simulink model "complex_iq_bw2048_out32_fixaxi_v12b"  (R2019a) (continued)
== system complex_iq_bw2048_out32_fixaxi_v12b/dec_fir1 == (continued)
  fir_dbl_col2/22(imag_back_out3) -> fir_dbl_col1/22(imag_back3)
  fir_dbl_col2/23(real_back_out4) -> fir_dbl_col1/23(real_back4)
  fir_dbl_col2/24(imag_back_out4) -> fir_dbl_col1/24(imag_back4)
  fir_dbl_col2/25(real_back_out5) -> fir_dbl_col1/25(real_back5)
  fir_dbl_col2/26(imag_back_out5) -> fir_dbl_col1/26(imag_back5)
  fir_dbl_col2/27(real_back_out6) -> fir_dbl_col1/27(real_back6)
  fir_dbl_col2/28(imag_back_out6) -> fir_dbl_col1/28(imag_back6)
  fir_dbl_col2/29(real_back_out7) -> fir_dbl_col1/29(real_back7)
  fir_dbl_col2/30(imag_back_out7) -> fir_dbl_col1/30(imag_back7)
  fir_dbl_col2/31(real_back_out8) -> fir_dbl_col1/31(real_back8)
  fir_dbl_col2/32(imag_back_out8) -> fir_dbl_col1/32(imag_back8)
  fir_dbl_col3/17(real_back_out1) -> fir_dbl_col2/17(real_back1)
  fir_dbl_col3/18(imag_back_out1) -> fir_dbl_col2/18(imag_back1)
  fir_dbl_col3/19(real_back_out2) -> fir_dbl_col2/19(real_back2)
  fir_dbl_col3/20(imag_back_out2) -> fir_dbl_col2/20(imag_back2)
  fir_dbl_col3/21(real_back_out3) -> fir_dbl_col2/21(real_back3)
  fir_dbl_col3/22(imag_back_out3) -> fir_dbl_col2/22(imag_back3)
  fir_dbl_col3/23(real_back_out4) -> fir_dbl_col2/23(real_back4)
  fir_dbl_col3/24(imag_back_out4) -> fir_dbl_col2/24(imag_back4)
  fir_dbl_col3/25(real_back_out5) -> fir_dbl_col2/25(real_back5)
  fir_dbl_col3/26(imag_back_out5) -> fir_dbl_col2/26(imag_back5)
  fir_dbl_col3/27(real_back_out6) -> fir_dbl_col2/27(real_back6)
  fir_dbl_col3/28(imag_back_out6) -> fir_dbl_col2/28(imag_back6)
  fir_dbl_col3/29(real_back_out7) -> fir_dbl_col2/29(real_back7)
  fir_dbl_col3/30(imag_back_out7) -> fir_dbl_col2/30(imag_back7)
  fir_dbl_col3/31(real_back_out8) -> fir_dbl_col2/31(real_back8)
  fir_dbl_col3/32(imag_back_out8) -> fir_dbl_col2/32(imag_back8)
  fir_dbl_col4/17(real_back_out1) -> fir_dbl_col3/17(real_back1)
  fir_dbl_col4/18(imag_back_out1) -> fir_dbl_col3/18(imag_back1)
  fir_dbl_col4/19(real_back_out2) -> fir_dbl_col3/19(real_back2)
  fir_dbl_col4/20(imag_back_out2) -> fir_dbl_col3/20(imag_back2)
  fir_dbl_col4/21(real_back_out3) -> fir_dbl_col3/21(real_back3)
  fir_dbl_col4/22(imag_back_out3) -> fir_dbl_col3/22(imag_back3)
  fir_dbl_col4/23(real_back_out4) -> fir_dbl_col3/23(real_back4)
  fir_dbl_col4/24(imag_back_out4) -> fir_dbl_col3/24(imag_back4)
  fir_dbl_col4/25(real_back_out5) -> fir_dbl_col3/25(real_back5)
  fir_dbl_col4/26(imag_back_out5) -> fir_dbl_col3/26(imag_back5)
  fir_dbl_col4/27(real_back_out6) -> fir_dbl_col3/27(real_back6)
  fir_dbl_col4/28(imag_back_out6) -> fir_dbl_col3/28(imag_back6)
  fir_dbl_col4/29(real_back_out7) -> fir_dbl_col3/29(real_back7)
  fir_dbl_col4/30(imag_back_out7) -> fir_dbl_col3/30(imag_back7)
  fir_dbl_col4/31(real_back_out8) -> fir_dbl_col3/31(real_back8)
  fir_dbl_col4/32(imag_back_out8) -> fir_dbl_col3/32(imag_back8)
  fir_dbl_col5/17(real_back_out1) -> fir_dbl_col4/17(real_back1)
  fir_dbl_col5/18(imag_back_out1) -> fir_dbl_col4/18(imag_back1)
  fir_dbl_col5/19(real_back_out2) -> fir_dbl_col4/19(real_back2)
  fir_dbl_col5/20(imag_back_out2) -> fir_dbl_col4/20(imag_back2)
  fir_dbl_col5/21(real_back_out3) -> fir_dbl_col4/21(real_back3)
  fir_dbl_col5/22(imag_back_out3) -> fir_dbl_col4/22(imag_back3)
  fir_dbl_col5/23(real_back_out4) -> fir_dbl_col4/23(real_back4)
  fir_dbl_col5/24(imag_back_out4) -> fir_dbl_col4/24(imag_back4)
  fir_dbl_col5/25(real_back_out5) -> fir_dbl_col4/25(real_back5)
  fir_dbl_col5/26(imag_back_out5) -> fir_dbl_col4/26(imag_back5)
  fir_dbl_col5/27(real_back_out6) -> fir_dbl_col4/27(real_back6)
  fir_dbl_col5/28(imag_back_out6) -> fir_dbl_col4/28(imag_back6)
  fir_dbl_col5/29(real_back_out7) -> fir_dbl_col4/29(real_back7)
  fir_dbl_col5/30(imag_back_out7) -> fir_dbl_col4/30(imag_back7)
  fir_dbl_col5/31(real_back_out8) -> fir_dbl_col4/31(real_back8)
  fir_dbl_col5/32(imag_back_out8) -> fir_dbl_col4/32(imag_back8)
  fir_dbl_col6/17(real_back_out1) -> fir_dbl_col5/17(real_back1)
  fir_dbl_col6/18(imag_back_out1) -> fir_dbl_col5/18(imag_back1)
  fir_dbl_col6/19(real_back_out2) -> fir_dbl_col5/19(real_back2)
  fir_dbl_col6/20(imag_back_out2) -> fir_dbl_col5/20(imag_back2)
  fir_dbl_col6/21(real_back_out3) -> fir_dbl_col5/21(real_back3)
  fir_dbl_col6/22(imag_back_out3) -> fir_dbl_col5/22(imag_back3)
  fir_dbl_col6/23(real_back_out4) -> fir_dbl_col5/23(real_back4)
  fir_dbl_col6/24(imag_back_out4) -> fir_dbl_col5/24(imag_back4)
  fir_dbl_col6/25(real_back_out5) -> fir_dbl_col5/25(real_back5)
  fir_dbl_col6/26(imag_back_out5) -> fir_dbl_col5/26(imag_back5)
  fir_dbl_col6/27(real_back_out6) -> fir_dbl_col5/27(real_back6)
  fir_dbl_col6/28(imag_back_out6) -> fir_dbl_col5/28(imag_back6)
  fir_dbl_col6/29(real_back_out7) -> fir_dbl_col5/29(real_back7)
  fir_dbl_col6/30(imag_back_out7) -> fir_dbl_col5/30(imag_back7)
  fir_dbl_col6/31(real_back_out8) -> fir_dbl_col5/31(real_back8)
  fir_dbl_col6/32(imag_back_out8) -> fir_dbl_col5/32(imag_back8)
  fir_dbl_col7/17(real_back_out1) -> fir_dbl_col6/17(real_back1)
  fir_dbl_col7/18(imag_back_out1) -> fir_dbl_col6/18(imag_back1)
  fir_dbl_col7/19(real_back_out2) -> fir_dbl_col6/19(real_back2)
  fir_dbl_col7/20(imag_back_out2) -> fir_dbl_col6/20(imag_back2)
  fir_dbl_col7/21(real_back_out3) -> fir_dbl_col6/21(real_back3)
  fir_dbl_col7/22(imag_back_out3) -> fir_dbl_col6/22(imag_back3)
  fir_dbl_col7/23(real_back_out4) -> fir_dbl_col6/23(real_back4)
  fir_dbl_col7/24(imag_back_out4) -> fir_dbl_col6/24(imag_back4)
  fir_dbl_col7/25(real_back_out5) -> fir_dbl_col6/25(real_back5)
  fir_dbl_col7/26(imag_back_out5) -> fir_dbl_col6/26(imag_back5)
  fir_dbl_col7/27(real_back_out6) -> fir_dbl_col6/27(real_back6)
  fir_dbl_col7/28(imag_back_out6) -> fir_dbl_col6/28(imag_back6)
  fir_dbl_col7/29(real_back_out7) -> fir_dbl_col6/29(real_back7)
  fir_dbl_col7/30(imag_back_out7) -> fir_dbl_col6/30(imag_back7)
  fir_dbl_col7/31(real_back_out8) -> fir_dbl_col6/31(real_back8)
  fir_dbl_col7/32(imag_back_out8) -> fir_dbl_col6/32(imag_back8)
  fir_dbl_col8/17(real_back_out1) -> fir_dbl_col7/17(real_back1)
  fir_dbl_col8/18(imag_back_out1) -> fir_dbl_col7/18(imag_back1)
  fir_dbl_col8/19(real_back_out2) -> fir_dbl_col7/19(real_back2)
  fir_dbl_col8/20(imag_back_out2) -> fir_dbl_col7/20(imag_back2)
  fir_dbl_col8/21(real_back_out3) -> fir_dbl_col7/21(real_back3)
  fir_dbl_col8/22(imag_back_out3) -> fir_dbl_col7/22(imag_back3)
  fir_dbl_col8/23(real_back_out4) -> fir_dbl_col7/23(real_back4)
  fir_dbl_col8/24(imag_back_out4) -> fir_dbl_col7/24(imag_back4)
  fir_dbl_col8/25(real_back_out5) -> fir_dbl_col7/25(real_back5)
  fir_dbl_col8/26(imag_back_out5) -> fir_dbl_col7/26(imag_back5)
  fir_dbl_col8/27(real_back_out6) -> fir_dbl_col7/27(real_back6)
  fir_dbl_col8/28(imag_back_out6) -> fir_dbl_col7/28(imag_back6)
  fir_dbl_col8/29(real_back_out7) -> fir_dbl_col7/29(real_back7)
  fir_dbl_col8/30(imag_back_out7) -> fir_dbl_col7/30(imag_back7)
  fir_dbl_col8/31(real_back_out8) -> fir_dbl_col7/31(real_back8)
  fir_dbl_col8/32(imag_back_out8) -> fir_dbl_col7/32(imag_back8)
  fir_dbl_col9/17(real_back_out1) -> fir_dbl_col8/17(real_back1)
  fir_dbl_col9/18(imag_back_out1) -> fir_dbl_col8/18(imag_back1)
  fir_dbl_col9/19(real_back_out2) -> fir_dbl_col8/19(real_back2)
  fir_dbl_col9/20(imag_back_out2) -> fir_dbl_col8/20(imag_back2)
  fir_dbl_col9/21(real_back_out3) -> fir_dbl_col8/21(real_back3)
  fir_dbl_col9/22(imag_back_out3) -> fir_dbl_col8/22(imag_back3)
  fir_dbl_col9/23(real_back_out4) -> fir_dbl_col8/23(real_back4)
  fir_dbl_col9/24(imag_back_out4) -> fir_dbl_col8/24(imag_back4)
  fir_dbl_col9/25(real_back_out5) -> fir_dbl_col8/25(real_back5)
  fir_dbl_col9/26(imag_back_out5) -> fir_dbl_col8/26(imag_back5)
  fir_dbl_col9/27(real_back_out6) -> fir_dbl_col8/27(real_back6)
  fir_dbl_col9/28(imag_back_out6) -> fir_dbl_col8/28(imag_back6)
  fir_dbl_col9/29(real_back_out7) -> fir_dbl_col8/29(real_back7)
  fir_dbl_col9/30(imag_back_out7) -> fir_dbl_col8/30(imag_back7)
  fir_dbl_col9/31(real_back_out8) -> fir_dbl_col8/31(real_back8)
  fir_dbl_col9/32(imag_back_out8) -> fir_dbl_col8/32(imag_back8)
  fir_dbl_col10/17(real_back_out1) -> fir_dbl_col9/17(real_back1)
  fir_dbl_col10/18(imag_back_out1) -> fir_dbl_col9/18(imag_back1)
  fir_dbl_col10/19(real_back_out2) -> fir_dbl_col9/19(real_back2)
  fir_dbl_col10/20(imag_back_out2) -> fir_dbl_col9/20(imag_back2)
  fir_dbl_col10/21(real_back_out3) -> fir_dbl_col9/21(real_back3)
  fir_dbl_col10/22(imag_back_out3) -> fir_dbl_col9/22(imag_back3)
  fir_dbl_col10/23(real_back_out4) -> fir_dbl_col9/23(real_back4)
  fir_dbl_col10/24(imag_back_out4) -> fir_dbl_col9/24(imag_back4)
  fir_dbl_col10/25(real_back_out5) -> fir_dbl_col9/25(real_back5)
  fir_dbl_col10/26(imag_back_out5) -> fir_dbl_col9/26(imag_back5)
  fir_dbl_col10/27(real_back_out6) -> fir_dbl_col9/27(real_back6)
  fir_dbl_col10/28(imag_back_out6) -> fir_dbl_col9/28(imag_back6)
  fir_dbl_col10/29(real_back_out7) -> fir_dbl_col9/29(real_back7)
  fir_dbl_col10/30(imag_back_out7) -> fir_dbl_col9/30(imag_back7)
  fir_dbl_col10/31(real_back_out8) -> fir_dbl_col9/31(real_back8)
  fir_dbl_col10/32(imag_back_out8) -> fir_dbl_col9/32(imag_back8)
  fir_dbl_col11/17(real_back_out1) -> fir_dbl_col10/17(real_back1)
  fir_dbl_col11/18(imag_back_out1) -> fir_dbl_col10/18(imag_back1)
  fir_dbl_col11/19(real_back_out2) -> fir_dbl_col10/19(real_back2)
  fir_dbl_col11/20(imag_back_out2) -> fir_dbl_col10/20(imag_back2)
  fir_dbl_col11/21(real_back_out3) -> fir_dbl_col10/21(real_back3)
  fir_dbl_col11/22(imag_back_out3) -> fir_dbl_col10/22(imag_back3)
  fir_dbl_col11/23(real_back_out4) -> fir_dbl_col10/23(real_back4)
  fir_dbl_col11/24(imag_back_out4) -> fir_dbl_col10/24(imag_back4)
  fir_dbl_col11/25(real_back_out5) -> fir_dbl_col10/25(real_back5)
  fir_dbl_col11/26(imag_back_out5) -> fir_dbl_col10/26(imag_back5)
  fir_dbl_col11/27(real_back_out6) -> fir_dbl_col10/27(real_back6)
  fir_dbl_col11/28(imag_back_out6) -> fir_dbl_col10/28(imag_back6)
  fir_dbl_col11/29(real_back_out7) -> fir_dbl_col10/29(real_back7)
  fir_dbl_col11/30(imag_back_out7) -> fir_dbl_col10/30(imag_back7)
  fir_dbl_col11/31(real_back_out8) -> fir_dbl_col10/31(real_back8)
  fir_dbl_col11/32(imag_back_out8) -> fir_dbl_col10/32(imag_back8)
  fir_dbl_col12/17(real_back_out1) -> fir_dbl_col11/17(real_back1)
  fir_dbl_col12/18(imag_back_out1) -> fir_dbl_col11/18(imag_back1)
  fir_dbl_col12/19(real_back_out2) -> fir_dbl_col11/19(real_back2)
  fir_dbl_col12/20(imag_back_out2) -> fir_dbl_col11/20(imag_back2)
  fir_dbl_col12/21(real_back_out3) -> fir_dbl_col11/21(real_back3)
  fir_dbl_col12/22(imag_back_out3) -> fir_dbl_col11/22(imag_back3)
  fir_dbl_col12/23(real_back_out4) -> fir_dbl_col11/23(real_back4)
  fir_dbl_col12/24(imag_back_out4) -> fir_dbl_col11/24(imag_back4)
  fir_dbl_col12/25(real_back_out5) -> fir_dbl_col11/25(real_back5)
  fir_dbl_col12/26(imag_back_out5) -> fir_dbl_col11/26(imag_back5)
  fir_dbl_col12/27(real_back_out6) -> fir_dbl_col11/27(real_back6)
  fir_dbl_col12/28(imag_back_out6) -> fir_dbl_col11/28(imag_back6)
  fir_dbl_col12/29(real_back_out7) -> fir_dbl_col11/29(real_back7)
  fir_dbl_col12/30(imag_back_out7) -> fir_dbl_col11/30(imag_back7)
  fir_dbl_col12/31(real_back_out8) -> fir_dbl_col11/31(real_back8)
  fir_dbl_col12/32(imag_back_out8) -> fir_dbl_col11/32(imag_back8)
  fir_dbl_col13/17(real_back_out1) -> fir_dbl_col12/17(real_back1)
  fir_dbl_col13/18(imag_back_out1) -> fir_dbl_col12/18(imag_back1)
  fir_dbl_col13/19(real_back_out2) -> fir_dbl_col12/19(real_back2)
  fir_dbl_col13/20(imag_back_out2) -> fir_dbl_col12/20(imag_back2)
  fir_dbl_col13/21(real_back_out3) -> fir_dbl_col12/21(real_back3)
  fir_dbl_col13/22(imag_back_out3) -> fir_dbl_col12/22(imag_back3)
  fir_dbl_col13/23(real_back_out4) -> fir_dbl_col12/23(real_back4)
  fir_dbl_col13/24(imag_back_out4) -> fir_dbl_col12/24(imag_back4)
  fir_dbl_col13/25(real_back_out5) -> fir_dbl_col12/25(real_back5)
  fir_dbl_col13/26(imag_back_out5) -> fir_dbl_col12/26(imag_back5)
  fir_dbl_col13/27(real_back_out6) -> fir_dbl_col12/27(real_back6)
  fir_dbl_col13/28(imag_back_out6) -> fir_dbl_col12/28(imag_back6)
  fir_dbl_col13/29(real_back_out7) -> fir_dbl_col12/29(real_back7)
  fir_dbl_col13/30(imag_back_out7) -> fir_dbl_col12/30(imag_back7)
  fir_dbl_col13/31(real_back_out8) -> fir_dbl_col12/31(real_back8)
  fir_dbl_col13/32(imag_back_out8) -> fir_dbl_col12/32(imag_back8)
  fir_dbl_col14/17(real_back_out1) -> fir_dbl_col13/17(real_back1)
  fir_dbl_col14/18(imag_back_out1) -> fir_dbl_col13/18(imag_back1)
  fir_dbl_col14/19(real_back_out2) -> fir_dbl_col13/19(real_back2)
  fir_dbl_col14/20(imag_back_out2) -> fir_dbl_col13/20(imag_back2)
  fir_dbl_col14/21(real_back_out3) -> fir_dbl_col13/21(real_back3)
  fir_dbl_col14/22(imag_back_out3) -> fir_dbl_col13/22(imag_back3)
  fir_dbl_col14/23(real_back_out4) -> fir_dbl_col13/23(real_back4)
  fir_dbl_col14/24(imag_back_out4) -> fir_dbl_col13/24(imag_back4)
  fir_dbl_col14/25(real_back_out5) -> fir_dbl_col13/25(real_back5)
  fir_dbl_col14/26(imag_back_out5) -> fir_dbl_col13/26(imag_back5)
  fir_dbl_col14/27(real_back_out6) -> fir_dbl_col13/27(real_back6)
  fir_dbl_col14/28(imag_back_out6) -> fir_dbl_col13/28(imag_back6)
  fir_dbl_col14/29(real_back_out7) -> fir_dbl_col13/29(real_back7)
  fir_dbl_col14/30(imag_back_out7) -> fir_dbl_col13/30(imag_back7)
  fir_dbl_col14/31(real_back_out8) -> fir_dbl_col13/31(real_back8)
  fir_dbl_col14/32(imag_back_out8) -> fir_dbl_col13/32(imag_back8)
  fir_dbl_col15/17(real_back_out1) -> fir_dbl_col14/17(real_back1)
  fir_dbl_col15/18(imag_back_out1) -> fir_dbl_col14/18(imag_back1)
  fir_dbl_col15/19(real_back_out2) -> fir_dbl_col14/19(real_back2)
  fir_dbl_col15/20(imag_back_out2) -> fir_dbl_col14/20(imag_back2)
  fir_dbl_col15/21(real_back_out3) -> fir_dbl_col14/21(real_back3)
  fir_dbl_col15/22(imag_back_out3) -> fir_dbl_col14/22(imag_back3)
  fir_dbl_col15/23(real_back_out4) -> fir_dbl_col14/23(real_back4)
  fir_dbl_col15/24(imag_back_out4) -> fir_dbl_col14/24(imag_back4)
  fir_dbl_col15/25(real_back_out5) -> fir_dbl_col14/25(real_back5)
  fir_dbl_col15/26(imag_back_out5) -> fir_dbl_col14/26(imag_back5)
  fir_dbl_col15/27(real_back_out6) -> fir_dbl_col14/27(real_back6)
  fir_dbl_col15/28(imag_back_out6) -> fir_dbl_col14/28(imag_back6)
  fir_dbl_col15/29(real_back_out7) -> fir_dbl_col14/29(real_back7)
  fir_dbl_col15/30(imag_back_out7) -> fir_dbl_col14/30(imag_back7)
  fir_dbl_col15/31(real_back_out8) -> fir_dbl_col14/31(real_back8)
  fir_dbl_col15/32(imag_back_out8) -> fir_dbl_col14/32(imag_back8)
  fir_dbl_col16/17(real_back_out1) -> fir_dbl_col15/17(real_back1)
  fir_dbl_col16/18(imag_back_out1) -> fir_dbl_col15/18(imag_back1)
  fir_dbl_col16/19(real_back_out2) -> fir_dbl_col15/19(real_back2)
  fir_dbl_col16/20(imag_back_out2) -> fir_dbl_col15/20(imag_back2)
  fir_dbl_col16/21(real_back_out3) -> fir_dbl_col15/21(real_back3)
  fir_dbl_col16/22(imag_back_out3) -> fir_dbl_col15/22(imag_back3)
  fir_dbl_col16/23(real_back_out4) -> fir_dbl_col15/23(real_back4)
  fir_dbl_col16/24(imag_back_out4) -> fir_dbl_col15/24(imag_back4)
  fir_dbl_col16/25(real_back_out5) -> fir_dbl_col15/25(real_back5)
  fir_dbl_col16/26(imag_back_out5) -> fir_dbl_col15/26(imag_back5)
  fir_dbl_col16/27(real_back_out6) -> fir_dbl_col15/27(real_back6)
  fir_dbl_col16/28(imag_back_out6) -> fir_dbl_col15/28(imag_back6)
  fir_dbl_col16/29(real_back_out7) -> fir_dbl_col15/29(real_back7)
  fir_dbl_col16/30(imag_back_out7) -> fir_dbl_col15/30(imag_back7)
  fir_dbl_col16/31(real_back_out8) -> fir_dbl_col15/31(real_back8)
  fir_dbl_col16/32(imag_back_out8) -> fir_dbl_col15/32(imag_back8)
  fir_dbl_col17/17(real_back_out1) -> fir_dbl_col16/17(real_back1)
  fir_dbl_col17/18(imag_back_out1) -> fir_dbl_col16/18(imag_back1)
  fir_dbl_col17/19(real_back_out2) -> fir_dbl_col16/19(real_back2)
  fir_dbl_col17/20(imag_back_out2) -> fir_dbl_col16/20(imag_back2)
  fir_dbl_col17/21(real_back_out3) -> fir_dbl_col16/21(real_back3)
  fir_dbl_col17/22(imag_back_out3) -> fir_dbl_col16/22(imag_back3)
  fir_dbl_col17/23(real_back_out4) -> fir_dbl_col16/23(real_back4)
  fir_dbl_col17/24(imag_back_out4) -> fir_dbl_col16/24(imag_back4)
  fir_dbl_col17/25(real_back_out5) -> fir_dbl_col16/25(real_back5)
  fir_dbl_col17/26(imag_back_out5) -> fir_dbl_col16/26(imag_back5)
  fir_dbl_col17/27(real_back_out6) -> fir_dbl_col16/27(real_back6)
  fir_dbl_col17/28(imag_back_out6) -> fir_dbl_col16/28(imag_back6)
  fir_dbl_col17/29(real_back_out7) -> fir_dbl_col16/29(real_back7)
  fir_dbl_col17/30(imag_back_out7) -> fir_dbl_col16/30(imag_back7)
  fir_dbl_col17/31(real_back_out8) -> fir_dbl_col16/31(real_back8)
  fir_dbl_col17/32(imag_back_out8) -> fir_dbl_col16/32(imag_back8)
  fir_dbl_col18/17(real_back_out1) -> fir_dbl_col17/17(real_back1)
  fir_dbl_col18/18(imag_back_out1) -> fir_dbl_col17/18(imag_back1)
  fir_dbl_col18/19(real_back_out2) -> fir_dbl_col17/19(real_back2)
  fir_dbl_col18/20(imag_back_out2) -> fir_dbl_col17/20(imag_back2)
  fir_dbl_col18/21(real_back_out3) -> fir_dbl_col17/21(real_back3)
  fir_dbl_col18/22(imag_back_out3) -> fir_dbl_col17/22(imag_back3)
  fir_dbl_col18/23(real_back_out4) -> fir_dbl_col17/23(real_back4)
  fir_dbl_col18/24(imag_back_out4) -> fir_dbl_col17/24(imag_back4)
  fir_dbl_col18/25(real_back_out5) -> fir_dbl_col17/25(real_back5)
  fir_dbl_col18/26(imag_back_out5) -> fir_dbl_col17/26(imag_back5)
  fir_dbl_col18/27(real_back_out6) -> fir_dbl_col17/27(real_back6)
  fir_dbl_col18/28(imag_back_out6) -> fir_dbl_col17/28(imag_back6)
  fir_dbl_col18/29(real_back_out7) -> fir_dbl_col17/29(real_back7)
  fir_dbl_col18/30(imag_back_out7) -> fir_dbl_col17/30(imag_back7)
  fir_dbl_col18/31(real_back_out8) -> fir_dbl_col17/31(real_back8)
  fir_dbl_col18/32(imag_back_out8) -> fir_dbl_col17/32(imag_back8)
  fir_dbl_col19/17(real_back_out1) -> fir_dbl_col18/17(real_back1)
  fir_dbl_col19/18(imag_back_out1) -> fir_dbl_col18/18(imag_back1)
  fir_dbl_col19/19(real_back_out2) -> fir_dbl_col18/19(real_back2)
  fir_dbl_col19/20(imag_back_out2) -> fir_dbl_col18/20(imag_back2)
  fir_dbl_col19/21(real_back_out3) -> fir_dbl_col18/21(real_back3)
  fir_dbl_col19/22(imag_back_out3) -> fir_dbl_col18/22(imag_back3)
  fir_dbl_col19/23(real_back_out4) -> fir_dbl_col18/23(real_back4)
  fir_dbl_col19/24(imag_back_out4) -> fir_dbl_col18/24(imag_back4)
  fir_dbl_col19/25(real_back_out5) -> fir_dbl_col18/25(real_back5)
  fir_dbl_col19/26(imag_back_out5) -> fir_dbl_col18/26(imag_back5)
  fir_dbl_col19/27(real_back_out6) -> fir_dbl_col18/27(real_back6)
  fir_dbl_col19/28(imag_back_out6) -> fir_dbl_col18/28(imag_back6)
  fir_dbl_col19/29(real_back_out7) -> fir_dbl_col18/29(real_back7)
  fir_dbl_col19/30(imag_back_out7) -> fir_dbl_col18/30(imag_back7)
  fir_dbl_col19/31(real_back_out8) -> fir_dbl_col18/31(real_back8)
  fir_dbl_col19/32(imag_back_out8) -> fir_dbl_col18/32(imag_back8)
  fir_dbl_col20/17(real_back_out1) -> fir_dbl_col19/17(real_back1)
  fir_dbl_col20/18(imag_back_out1) -> fir_dbl_col19/18(imag_back1)
  fir_dbl_col20/19(real_back_out2) -> fir_dbl_col19/19(real_back2)
  fir_dbl_col20/20(imag_back_out2) -> fir_dbl_col19/20(imag_back2)
  fir_dbl_col20/21(real_back_out3) -> fir_dbl_col19/21(real_back3)
  fir_dbl_col20/22(imag_back_out3) -> fir_dbl_col19/22(imag_back3)
  fir_dbl_col20/23(real_back_out4) -> fir_dbl_col19/23(real_back4)
  fir_dbl_col20/24(imag_back_out4) -> fir_dbl_col19/24(imag_back4)
  fir_dbl_col20/25(real_back_out5) -> fir_dbl_col19/25(real_back5)
  fir_dbl_col20/26(imag_back_out5) -> fir_dbl_col19/26(imag_back5)
  fir_dbl_col20/27(real_back_out6) -> fir_dbl_col19/27(real_back6)
  fir_dbl_col20/28(imag_back_out6) -> fir_dbl_col19/28(imag_back6)
  fir_dbl_col20/29(real_back_out7) -> fir_dbl_col19/29(real_back7)
  fir_dbl_col20/30(imag_back_out7) -> fir_dbl_col19/30(imag_back7)
  fir_dbl_col20/31(real_back_out8) -> fir_dbl_col19/31(real_back8)
  fir_dbl_col20/32(imag_back_out8) -> fir_dbl_col19/32(imag_back8)
  fir_dbl_col21/17(real_back_out1) -> fir_dbl_col20/17(real_back1)
  fir_dbl_col21/18(imag_back_out1) -> fir_dbl_col20/18(imag_back1)
  fir_dbl_col21/19(real_back_out2) -> fir_dbl_col20/19(real_back2)
  fir_dbl_col21/20(imag_back_out2) -> fir_dbl_col20/20(imag_back2)
  fir_dbl_col21/21(real_back_out3) -> fir_dbl_col20/21(real_back3)
  fir_dbl_col21/22(imag_back_out3) -> fir_dbl_col20/22(imag_back3)
  fir_dbl_col21/23(real_back_out4) -> fir_dbl_col20/23(real_back4)
  fir_dbl_col21/24(imag_back_out4) -> fir_dbl_col20/24(imag_back4)
  fir_dbl_col21/25(real_back_out5) -> fir_dbl_col20/25(real_back5)
  fir_dbl_col21/26(imag_back_out5) -> fir_dbl_col20/26(imag_back5)
  fir_dbl_col21/27(real_back_out6) -> fir_dbl_col20/27(real_back6)
  fir_dbl_col21/28(imag_back_out6) -> fir_dbl_col20/28(imag_back6)
  fir_dbl_col21/29(real_back_out7) -> fir_dbl_col20/29(real_back7)
  fir_dbl_col21/30(imag_back_out7) -> fir_dbl_col20/30(imag_back7)
  fir_dbl_col21/31(real_back_out8) -> fir_dbl_col20/31(real_back8)
  fir_dbl_col21/32(imag_back_out8) -> fir_dbl_col20/32(imag_back8)
  fir_dbl_col22/17(real_back_out1) -> fir_dbl_col21/17(real_back1)
  fir_dbl_col22/18(imag_back_out1) -> fir_dbl_col21/18(imag_back1)
  fir_dbl_col22/19(real_back_out2) -> fir_dbl_col21/19(real_back2)
  fir_dbl_col22/20(imag_back_out2) -> fir_dbl_col21/20(imag_back2)
  fir_dbl_col22/21(real_back_out3) -> fir_dbl_col21/21(real_back3)
  fir_dbl_col22/22(imag_back_out3) -> fir_dbl_col21/22(imag_back3)
  fir_dbl_col22/23(real_back_out4) -> fir_dbl_col21/23(real_back4)
  fir_dbl_col22/24(imag_back_out4) -> fir_dbl_col21/24(imag_back4)
  fir_dbl_col22/25(real_back_out5) -> fir_dbl_col21/25(real_back5)
  fir_dbl_col22/26(imag_back_out5) -> fir_dbl_col21/26(imag_back5)
  fir_dbl_col22/27(real_back_out6) -> fir_dbl_col21/27(real_back6)
  fir_dbl_col22/28(imag_back_out6) -> fir_dbl_col21/28(imag_back6)
  fir_dbl_col22/29(real_back_out7) -> fir_dbl_col21/29(real_back7)
  fir_dbl_col22/30(imag_back_out7) -> fir_dbl_col21/30(imag_back7)
  fir_dbl_col22/31(real_back_out8) -> fir_dbl_col21/31(real_back8)
  fir_dbl_col22/32(imag_back_out8) -> fir_dbl_col21/32(imag_back8)
  fir_dbl_col23/17(real_back_out1) -> fir_dbl_col22/17(real_back1)
  fir_dbl_col23/18(imag_back_out1) -> fir_dbl_col22/18(imag_back1)
  fir_dbl_col23/19(real_back_out2) -> fir_dbl_col22/19(real_back2)
  fir_dbl_col23/20(imag_back_out2) -> fir_dbl_col22/20(imag_back2)
  fir_dbl_col23/21(real_back_out3) -> fir_dbl_col22/21(real_back3)
  fir_dbl_col23/22(imag_back_out3) -> fir_dbl_col22/22(imag_back3)
  fir_dbl_col23/23(real_back_out4) -> fir_dbl_col22/23(real_back4)
  fir_dbl_col23/24(imag_back_out4) -> fir_dbl_col22/24(imag_back4)
  fir_dbl_col23/25(real_back_out5) -> fir_dbl_col22/25(real_back5)
  fir_dbl_col23/26(imag_back_out5) -> fir_dbl_col22/26(imag_back5)
  fir_dbl_col23/27(real_back_out6) -> fir_dbl_col22/27(real_back6)
  fir_dbl_col23/28(imag_back_out6) -> fir_dbl_col22/28(imag_back6)
  fir_dbl_col23/29(real_back_out7) -> fir_dbl_col22/29(real_back7)
  fir_dbl_col23/30(imag_back_out7) -> fir_dbl_col22/30(imag_back7)
  fir_dbl_col23/31(real_back_out8) -> fir_dbl_col22/31(real_back8)
  fir_dbl_col23/32(imag_back_out8) -> fir_dbl_col22/32(imag_back8)
  fir_dbl_col24/17(real_back_out1) -> fir_dbl_col23/17(real_back1)
  fir_dbl_col24/18(imag_back_out1) -> fir_dbl_col23/18(imag_back1)
  fir_dbl_col24/19(real_back_out2) -> fir_dbl_col23/19(real_back2)
  fir_dbl_col24/20(imag_back_out2) -> fir_dbl_col23/20(imag_back2)
  fir_dbl_col24/21(real_back_out3) -> fir_dbl_col23/21(real_back3)
  fir_dbl_col24/22(imag_back_out3) -> fir_dbl_col23/22(imag_back3)
  fir_dbl_col24/23(real_back_out4) -> fir_dbl_col23/23(real_back4)
  fir_dbl_col24/24(imag_back_out4) -> fir_dbl_col23/24(imag_back4)
  fir_dbl_col24/25(real_back_out5) -> fir_dbl_col23/25(real_back5)
  fir_dbl_col24/26(imag_back_out5) -> fir_dbl_col23/26(imag_back5)
  fir_dbl_col24/27(real_back_out6) -> fir_dbl_col23/27(real_back6)
  fir_dbl_col24/28(imag_back_out6) -> fir_dbl_col23/28(imag_back6)
  fir_dbl_col24/29(real_back_out7) -> fir_dbl_col23/29(real_back7)
  fir_dbl_col24/30(imag_back_out7) -> fir_dbl_col23/30(imag_back7)
  fir_dbl_col24/31(real_back_out8) -> fir_dbl_col23/31(real_back8)
  fir_dbl_col24/32(imag_back_out8) -> fir_dbl_col23/32(imag_back8)
  fir_dbl_col25/17(real_back_out1) -> fir_dbl_col24/17(real_back1)
  fir_dbl_col25/18(imag_back_out1) -> fir_dbl_col24/18(imag_back1)
  fir_dbl_col25/19(real_back_out2) -> fir_dbl_col24/19(real_back2)
  fir_dbl_col25/20(imag_back_out2) -> fir_dbl_col24/20(imag_back2)
  fir_dbl_col25/21(real_back_out3) -> fir_dbl_col24/21(real_back3)
  fir_dbl_col25/22(imag_back_out3) -> fir_dbl_col24/22(imag_back3)
  fir_dbl_col25/23(real_back_out4) -> fir_dbl_col24/23(real_back4)
  fir_dbl_col25/24(imag_back_out4) -> fir_dbl_col24/24(imag_back4)
  fir_dbl_col25/25(real_back_out5) -> fir_dbl_col24/25(real_back5)
  fir_dbl_col25/26(imag_back_out5) -> fir_dbl_col24/26(imag_back5)
  fir_dbl_col25/27(real_back_out6) -> fir_dbl_col24/27(real_back6)
  fir_dbl_col25/28(imag_back_out6) -> fir_dbl_col24/28(imag_back6)
  fir_dbl_col25/29(real_back_out7) -> fir_dbl_col24/29(real_back7)
  fir_dbl_col25/30(imag_back_out7) -> fir_dbl_col24/30(imag_back7)
  fir_dbl_col25/31(real_back_out8) -> fir_dbl_col24/31(real_back8)
  fir_dbl_col25/32(imag_back_out8) -> fir_dbl_col24/32(imag_back8)
  fir_dbl_col26/17(real_back_out1) -> fir_dbl_col25/17(real_back1)
  fir_dbl_col26/18(imag_back_out1) -> fir_dbl_col25/18(imag_back1)
  fir_dbl_col26/19(real_back_out2) -> fir_dbl_col25/19(real_back2)
  fir_dbl_col26/20(imag_back_out2) -> fir_dbl_col25/20(imag_back2)
  fir_dbl_col26/21(real_back_out3) -> fir_dbl_col25/21(real_back3)
  fir_dbl_col26/22(imag_back_out3) -> fir_dbl_col25/22(imag_back3)
  fir_dbl_col26/23(real_back_out4) -> fir_dbl_col25/23(real_back4)
  fir_dbl_col26/24(imag_back_out4) -> fir_dbl_col25/24(imag_back4)
  fir_dbl_col26/25(real_back_out5) -> fir_dbl_col25/25(real_back5)
  fir_dbl_col26/26(imag_back_out5) -> fir_dbl_col25/26(imag_back5)
  fir_dbl_col26/27(real_back_out6) -> fir_dbl_col25/27(real_back6)
  fir_dbl_col26/28(imag_back_out6) -> fir_dbl_col25/28(imag_back6)
  fir_dbl_col26/29(real_back_out7) -> fir_dbl_col25/29(real_back7)
  fir_dbl_col26/30(imag_back_out7) -> fir_dbl_col25/30(imag_back7)
  fir_dbl_col26/31(real_back_out8) -> fir_dbl_col25/31(real_back8)
  fir_dbl_col26/32(imag_back_out8) -> fir_dbl_col25/32(imag_back8)
  fir_dbl_col27/17(real_back_out1) -> fir_dbl_col26/17(real_back1)
  fir_dbl_col27/18(imag_back_out1) -> fir_dbl_col26/18(imag_back1)
  fir_dbl_col27/19(real_back_out2) -> fir_dbl_col26/19(real_back2)
  fir_dbl_col27/20(imag_back_out2) -> fir_dbl_col26/20(imag_back2)
  fir_dbl_col27/21(real_back_out3) -> fir_dbl_col26/21(real_back3)
  fir_dbl_col27/22(imag_back_out3) -> fir_dbl_col26/22(imag_back3)
  fir_dbl_col27/23(real_back_out4) -> fir_dbl_col26/23(real_back4)
  fir_dbl_col27/24(imag_back_out4) -> fir_dbl_col26/24(imag_back4)
  fir_dbl_col27/25(real_back_out5) -> fir_dbl_col26/25(real_back5)
  fir_dbl_col27/26(imag_back_out5) -> fir_dbl_col26/26(imag_back5)
  fir_dbl_col27/27(real_back_out6) -> fir_dbl_col26/27(real_back6)
  fir_dbl_col27/28(imag_back_out6) -> fir_dbl_col26/28(imag_back6)
  fir_dbl_col27/29(real_back_out7) -> fir_dbl_col26/29(real_back7)
  fir_dbl_col27/30(imag_back_out7) -> fir_dbl_col26/30(imag_back7)
  fir_dbl_col27/31(real_back_out8) -> fir_dbl_col26/31(real_back8)
  fir_dbl_col27/32(imag_back_out8) -> fir_dbl_col26/32(imag_back8)
  fir_dbl_col28/17(real_back_out1) -> fir_dbl_col27/17(real_back1)
  fir_dbl_col28/18(imag_back_out1) -> fir_dbl_col27/18(imag_back1)
  fir_dbl_col28/19(real_back_out2) -> fir_dbl_col27/19(real_back2)
  fir_dbl_col28/20(imag_back_out2) -> fir_dbl_col27/20(imag_back2)
  fir_dbl_col28/21(real_back_out3) -> fir_dbl_col27/21(real_back3)
  fir_dbl_col28/22(imag_back_out3) -> fir_dbl_col27/22(imag_back3)
  fir_dbl_col28/23(real_back_out4) -> fir_dbl_col27/23(real_back4)
  fir_dbl_col28/24(imag_back_out4) -> fir_dbl_col27/24(imag_back4)
  fir_dbl_col28/25(real_back_out5) -> fir_dbl_col27/25(real_back5)
  fir_dbl_col28/26(imag_back_out5) -> fir_dbl_col27/26(imag_back5)
  fir_dbl_col28/27(real_back_out6) -> fir_dbl_col27/27(real_back6)
  fir_dbl_col28/28(imag_back_out6) -> fir_dbl_col27/28(imag_back6)
  fir_dbl_col28/29(real_back_out7) -> fir_dbl_col27/29(real_back7)
  fir_dbl_col28/30(imag_back_out7) -> fir_dbl_col27/30(imag_back7)
  fir_dbl_col28/31(real_back_out8) -> fir_dbl_col27/31(real_back8)
  fir_dbl_col28/32(imag_back_out8) -> fir_dbl_col27/32(imag_back8)
  fir_dbl_col29/17(real_back_out1) -> fir_dbl_col28/17(real_back1)
  fir_dbl_col29/18(imag_back_out1) -> fir_dbl_col28/18(imag_back1)
  fir_dbl_col29/19(real_back_out2) -> fir_dbl_col28/19(real_back2)
  fir_dbl_col29/20(imag_back_out2) -> fir_dbl_col28/20(imag_back2)
  fir_dbl_col29/21(real_back_out3) -> fir_dbl_col28/21(real_back3)
  fir_dbl_col29/22(imag_back_out3) -> fir_dbl_col28/22(imag_back3)
  fir_dbl_col29/23(real_back_out4) -> fir_dbl_col28/23(real_back4)
  fir_dbl_col29/24(imag_back_out4) -> fir_dbl_col28/24(imag_back4)
  fir_dbl_col29/25(real_back_out5) -> fir_dbl_col28/25(real_back5)
  fir_dbl_col29/26(imag_back_out5) -> fir_dbl_col28/26(imag_back5)
  fir_dbl_col29/27(real_back_out6) -> fir_dbl_col28/27(real_back6)
  fir_dbl_col29/28(imag_back_out6) -> fir_dbl_col28/28(imag_back6)
  fir_dbl_col29/29(real_back_out7) -> fir_dbl_col28/29(real_back7)
  fir_dbl_col29/30(imag_back_out7) -> fir_dbl_col28/30(imag_back7)
  fir_dbl_col29/31(real_back_out8) -> fir_dbl_col28/31(real_back8)
  fir_dbl_col29/32(imag_back_out8) -> fir_dbl_col28/32(imag_back8)
  fir_dbl_col30/17(real_back_out1) -> fir_dbl_col29/17(real_back1)
  fir_dbl_col30/18(imag_back_out1) -> fir_dbl_col29/18(imag_back1)
  fir_dbl_col30/19(real_back_out2) -> fir_dbl_col29/19(real_back2)
  fir_dbl_col30/20(imag_back_out2) -> fir_dbl_col29/20(imag_back2)
  fir_dbl_col30/21(real_back_out3) -> fir_dbl_col29/21(real_back3)
  fir_dbl_col30/22(imag_back_out3) -> fir_dbl_col29/22(imag_back3)
  fir_dbl_col30/23(real_back_out4) -> fir_dbl_col29/23(real_back4)
  fir_dbl_col30/24(imag_back_out4) -> fir_dbl_col29/24(imag_back4)
  fir_dbl_col30/25(real_back_out5) -> fir_dbl_col29/25(real_back5)
  fir_dbl_col30/26(imag_back_out5) -> fir_dbl_col29/26(imag_back5)
  fir_dbl_col30/27(real_back_out6) -> fir_dbl_col29/27(real_back6)
  fir_dbl_col30/28(imag_back_out6) -> fir_dbl_col29/28(imag_back6)
  fir_dbl_col30/29(real_back_out7) -> fir_dbl_col29/29(real_back7)
  fir_dbl_col30/30(imag_back_out7) -> fir_dbl_col29/30(imag_back7)
  fir_dbl_col30/31(real_back_out8) -> fir_dbl_col29/31(real_back8)
  fir_dbl_col30/32(imag_back_out8) -> fir_dbl_col29/32(imag_back8)
  fir_dbl_col31/17(real_back_out1) -> fir_dbl_col30/17(real_back1)
  fir_dbl_col31/18(imag_back_out1) -> fir_dbl_col30/18(imag_back1)
  fir_dbl_col31/19(real_back_out2) -> fir_dbl_col30/19(real_back2)
  fir_dbl_col31/20(imag_back_out2) -> fir_dbl_col30/20(imag_back2)
  fir_dbl_col31/21(real_back_out3) -> fir_dbl_col30/21(real_back3)
  fir_dbl_col31/22(imag_back_out3) -> fir_dbl_col30/22(imag_back3)
  fir_dbl_col31/23(real_back_out4) -> fir_dbl_col30/23(real_back4)
  fir_dbl_col31/24(imag_back_out4) -> fir_dbl_col30/24(imag_back4)
  fir_dbl_col31/25(real_back_out5) -> fir_dbl_col30/25(real_back5)
  fir_dbl_col31/26(imag_back_out5) -> fir_dbl_col30/26(imag_back5)
  fir_dbl_col31/27(real_back_out6) -> fir_dbl_col30/27(real_back6)
  fir_dbl_col31/28(imag_back_out6) -> fir_dbl_col30/28(imag_back6)
  fir_dbl_col31/29(real_back_out7) -> fir_dbl_col30/29(real_back7)
  fir_dbl_col31/30(imag_back_out7) -> fir_dbl_col30/30(imag_back7)
  fir_dbl_col31/31(real_back_out8) -> fir_dbl_col30/31(real_back8)
  fir_dbl_col31/32(imag_back_out8) -> fir_dbl_col30/32(imag_back8)
  fir_dbl_col32/17(real_back_out1) -> fir_dbl_col31/17(real_back1)
  fir_dbl_col32/18(imag_back_out1) -> fir_dbl_col31/18(imag_back1)
  fir_dbl_col32/19(real_back_out2) -> fir_dbl_col31/19(real_back2)
  fir_dbl_col32/20(imag_back_out2) -> fir_dbl_col31/20(imag_back2)
  fir_dbl_col32/21(real_back_out3) -> fir_dbl_col31/21(real_back3)
  fir_dbl_col32/22(imag_back_out3) -> fir_dbl_col31/22(imag_back3)
  fir_dbl_col32/23(real_back_out4) -> fir_dbl_col31/23(real_back4)
  fir_dbl_col32/24(imag_back_out4) -> fir_dbl_col31/24(imag_back4)
  fir_dbl_col32/25(real_back_out5) -> fir_dbl_col31/25(real_back5)
  fir_dbl_col32/26(imag_back_out5) -> fir_dbl_col31/26(imag_back5)
  fir_dbl_col32/27(real_back_out6) -> fir_dbl_col31/27(real_back6)
  fir_dbl_col32/28(imag_back_out6) -> fir_dbl_col31/28(imag_back6)
  fir_dbl_col32/29(real_back_out7) -> fir_dbl_col31/29(real_back7)
  fir_dbl_col32/30(imag_back_out7) -> fir_dbl_col31/30(imag_back7)
  fir_dbl_col32/31(real_back_out8) -> fir_dbl_col31/31(real_back8)
  fir_dbl_col32/32(imag_back_out8) -> fir_dbl_col31/32(imag_back8)
  fir_dbl_col32/1(real_out1) -> fir_dbl_col32/17(real_back1)
  fir_dbl_col32/2(imag_out1) -> fir_dbl_col32/18(imag_back1)
  fir_dbl_col32/3(real_out2) -> fir_dbl_col32/19(real_back2)
  fir_dbl_col32/4(imag_out2) -> fir_dbl_col32/20(imag_back2)
  fir_dbl_col32/5(real_out3) -> fir_dbl_col32/21(real_back3)
  fir_dbl_col32/6(imag_out3) -> fir_dbl_col32/22(imag_back3)
  fir_dbl_col32/7(real_out4) -> fir_dbl_col32/23(real_back4)
  fir_dbl_col32/8(imag_out4) -> fir_dbl_col32/24(imag_back4)
  fir_dbl_col32/9(real_out5) -> fir_dbl_col32/25(real_back5)
  fir_dbl_col32/10(imag_out5) -> fir_dbl_col32/26(imag_back5)
  fir_dbl_col32/11(real_out6) -> fir_dbl_col32/27(real_back6)
  fir_dbl_col32/12(imag_out6) -> fir_dbl_col32/28(imag_back6)
  fir_dbl_col32/13(real_out7) -> fir_dbl_col32/29(real_back7)
  fir_dbl_col32/14(imag_out7) -> fir_dbl_col32/30(imag_back7)
  fir_dbl_col32/15(real_out8) -> fir_dbl_col32/31(real_back8)
  fir_dbl_col32/16(imag_out8) -> fir_dbl_col32/32(imag_back8)
